simulink model "v01_aa_rii_16r4t11f_ver118sa"  (Simulink 7.6)
settings: Solver=ode45  StartTime=0.0  StopTime=128000  RelTol=1e-3
undefined symbols (not defined in the file or in its folder): sfp
libraries: xbsIndex_r4 x26962, monroe_library x154, xbsReplacements_r4 x78, simulink x32, dspsigops x32, casper_library_misc x30, xps_library x18
(part 22 of 176)

== system v01_aa_rii_16r4t11f_ver118sa/pfb_fir_real2/coeff_gen_0_15 ==
  sync : Inport
  Counter0 : xbsIndex_r4/Counter  cnt_type=Free Running  cnt_to=Inf  operation=Up  start_count=8  cnt_by_val=1  arith_type=Unsigned  n_bits=7  bin_pt=0  load_pin=off  rst=on  en=off  explicit_period=on  period=1  dbl_ovrd=off  use_behavioral_HDL=off  implementation=Fabric  block_type=counter
  Counter1 : xbsIndex_r4/Counter  cnt_type=Free Running  cnt_to=Inf  operation=Down  start_count=119  cnt_by_val=1  arith_type=Unsigned  n_bits=7  bin_pt=0  load_pin=off  rst=on  en=off  explicit_period=on  period=1  dbl_ovrd=off  use_behavioral_HDL=off  implementation=Fabric  block_type=counter
  Delay1 : xbsIndex_r4/Delay  rst=off  infoeditControl=Selection of Reset will increase slice count due to use of real FFs and instead of SRLs  en=off  latency=2  dbl_ovrd=off  reg_retiming=off  block_type=delay
  Delay2 : xbsIndex_r4/Delay  rst=off  infoeditControl=Selection of Reset will increase slice count due to use of real FFs and instead of SRLs  en=off  latency=1  dbl_ovrd=off  reg_retiming=off  block_type=delay
  Delay3 : xbsIndex_r4/Delay  rst=off  infoeditControl=Selection of Reset will increase slice count due to use of real FFs and instead of SRLs  en=off  latency=1  dbl_ovrd=off  reg_retiming=off  block_type=delay
  Delay4 : xbsIndex_r4/Delay  rst=off  infoeditControl=Selection of Reset will increase slice count due to use of real FFs and instead of SRLs  en=off  latency=2  dbl_ovrd=off  reg_retiming=off  block_type=delay
  coeff_ram_1 : xbsIndex_r4/Dual Port RAM  depth=128  initVector=[-9.76681628083641e-06 -0.000323674631039594 -0.000640685368821483 -0.000961464852068701 -0.00128668595239865 -0.00161702698554985 -0.00195317007673584 -0.00229579949852553 -0.00264559998341877 -0.00300325501333463 -0.00336944508827481 -0.00374484597646818 -0.0041301269483421 -0.00452594899670393 -0.00493296304555074 -0.00535180814995662 -0.00578310968951604 -0.00622747755784734 -0.00668550435068305 -0.00715776355509352 -0.00764480774240668 -0.00814716676740016 -0.00866534597635216 -0.00919982442654445 -0.00975105311981421 -0.0103194532527527 -0.0109054144861447 -0.011509293236238 -0.0121314109904214 -0.0127720526498779 -0.0134314649017638 -0.0141098546234445 -0.0148073873212959 -0.015524185606554 -0.0162603277106669 -0.0170158460425702 -0.0177907257902727 -0.0185849035690979 -0.0193982661188903 -0.0202306490524425 -0.0210818356573608 -0.0219515557535275 -0.0228394846082689 -0.0237452419112808 -0.0246683908113017 -0.0256084370164626 -0.0265648279601778 -0.0275369520343712 -0.0285241378917642 -0.0295256538188765 -0.0305407071813169 -0.0315684439428629 -0.0326079482597467 -0.0336582421514863 -0.0347182852495099 -0.0357869746247431 -0.0368631446952324 -0.0379455672147943 -0.0390329513435835 -0.0401239438013823 -0.0412171291043174 -0.0423110298856138 -0.0434041073008984 -0.0444947615184662 -0.0455813322948223 -0.0466620996357123 -0.0477352845427513 -0.0487990498456597 -0.0498515011200101 -0.0508906876902881 -0.0519146037179613 -0.0529211893741533 -0.0539083320964084 -0.0548738679289332 -0.0558155829455944 -0.0567312147548497 -0.0576184540856848 -0.0584749464535256 -0.0592982939049929 -0.0600860568402664 -0.0608357559117222 -0.0615448739974078 -0.0622108582478242 -0.0628311222043803 -0.0634030479877959 -0.0639239885546298 -0.0643912700200192 -0.0648021940446247 -0.0651540402836856 -0.0654440688960025 -0.06566952311058 -0.0658276318485765 -0.0659156123981315 -0.0659306731395561 -0.0658700163183022 -0.0657308408630492 -0.0655103452461749 -0.0652057303838135 -0.0648142025726343 -0.0643329764604135 -0.0637592780474138 -0.0630903477155274 -0.0623234432820887 -0.0614558430752086 -0.0604848490274413 -0.0594077897845465 -0.0582220238260738 -0.056924942594458 -0.0555139736292838 -0.0539865837033466 -0.0523402819571135 -0.0505726230281672 -0.0486812101721953 -0.0466636983720764 -0.0445177974316036 -0.0422412750503798 -0.0398319598764152 -0.0372877445329644 -0.0346065886161369 -0.0317865216598359 -0.0288256460645821 -0.0257221399868061 -0.0224742601852073 -0.0190803448208065 -0.0155388162073467 -0.011848183508731 -0.00800704538021982 -0.00401409255015518]  distributed_mem=Block RAM  init_a=0  init_b=0  rst_a=off  rst_b=off  en_a=off  en_b=off  latency=2  write_mode_A=No Read On Write  write_mode_B=No Read On Write  dbl_ovrd=off  optimize=Area  block_type=dpram
  coeff_ram_2 : xbsIndex_r4/Dual Port RAM  depth=128  initVector=[0.000131889660979235 0.00443201888730544 0.00888731333319452 0.013498689460842 0.0182669597527502 0.0231928305520307 0.0282768999833737 0.0335196559574605 0.0389214742615229 0.0444826167386781 0.0502032295585852 0.0560833415818892 0.0621228628208312 0.0683215829983153 0.0746791702076299 0.0811951696749281 0.0878690026264726 0.0946999652625533 0.10168722783988 0.108829833864151 0.116126699394389 0.123576612460526 0.131178232595614 0.138930090483912 0.146830587726002 0.154877996721945 0.163070460673406 0.171405993705518 0.179882481109162 0.188497679704198 0.197249218324085 0.206134598422161 0.215151194799783 0.224296256456348 0.233566907561132 0.242960148546725 0.252472857323742 0.262101790616336 0.271843585417932 0.28169476056646 0.291651718438258 0.301710746759654 0.311868020535164 0.322119604091054 0.332461453232956 0.342889417516048 0.353399242626224 0.363986572870548 0.374646953775156 0.385375834788674 0.396168572089086 0.407020431491882 0.417926591457195 0.428882146193547 0.439882108855684 0.450921414833902 0.461994925132158 0.473097429832141 0.48422365164041 0.495368249515587 0.506525822372513 0.517690912860181 0.528858011210183 0.540021559152312 0.551175953893889 0.562315552159312 0.573434674286248 0.584527608374814 0.595588614486041 0.606611928885839 0.617591768330647 0.628522334390862 0.639397817808129 0.650212402882503 0.660960271885469 0.671635609494744 0.682232607246797 0.692745468002935 0.703168410424828 0.713495673455308 0.723721520800239 0.733840245407289 0.743846173937386 0.753733671224655 0.763497144720641 0.773131048918607 0.782629889753732 0.791988228975025 0.801200688484807 0.810261954641631 0.819166782522532 0.827910000140534 0.836486512613386 0.844891306279528 0.853119452757334 0.861166112943733 0.869026540948364 0.876696087959463 0.884170206037768 0.891444451834754 0.898514490231627 0.905376097895536 0.912025166749558 0.9184577073531 0.924669852189424 0.930657858857099 0.936418113162282 0.941947132108819 0.947241566783232 0.952298205131806 0.957113974627046 0.961685944820906 0.966011329782295 0.970087490416478 0.9739119366641 0.977482329577674 0.980796483273521 0.983852366757218 0.986648105620789 0.989181983609969 0.991452444059991 0.993458091198508 0.995197691314345 0.996670173790967 0.997874632003604 0.998810324079201 0.999476673518401 0.999873269678985]  distributed_mem=Block RAM  init_a=0  init_b=0  rst_a=off  rst_b=off  en_a=off  en_b=off  latency=2  write_mode_A=No Read On Write  write_mode_B=No Read On Write  dbl_ovrd=off  optimize=Area  block_type=dpram
  coeff_ram_3 : xbsIndex_r4/Dual Port RAM  depth=128  initVector=[0.999999868120266 0.999856390808123 0.999442926180455 0.998759729072997 0.997807220505575 0.996585987329003 0.995096781732979 0.993340520615446 0.99131828481406 0.989031318200503 0.986481026638539 0.983668976806834 0.980596894887697 0.977266665123027 0.973680328238889 0.969840079740251 0.96574826807758 0.961407392687059 0.956820101906379 0.95198919076813 0.946917598672946 0.941608406944702 0.936064836270128 0.930290244025361 0.924288121492039 0.918062090965654 0.91161590275898 0.904953432103512 0.898078675951906 0.890995749684561 0.883708883723521 0.876222420057003 0.868540808677901 0.860668603939747 0.852610460833631 0.844371131189694 0.835955459806863 0.827368380514556 0.818614912170158 0.809700154596117 0.800629284460566 0.791407551105419 0.782040272325951 0.772532830105885 0.762890666312066 0.753119278352835 0.743224214804217 0.733211071008104 0.723085484646579 0.712853131296598 0.702519719969201 0.692090988637482 0.681572699757496 0.670970635786334 0.660290594701535 0.649538385526024 0.638719823862754 0.627840727443177 0.616906911693676 0.605924185324051 0.5948983459421 0.583835175698334 0.572740436964789 0.561619868051874 0.550479178967135 0.539324047219761 0.528160113674606 0.516992978459435 0.50582819692903 0.494671275689747 0.48352766868801 0.472402773366174 0.461301926889109 0.45023040244476 0.439193405621868 0.428196070867941 0.417243458030464 0.406340548984262 0.39549224434781 0.384703360291205 0.373978625438387 0.363322677866115 0.35274006220209 0.342235226824484 0.331812521165061 0.321476193117935 0.311230386555891 0.301079138956108 0.291026379136963 0.281075925107508 0.271231482031073 0.26149664030433 0.25187487375303 0.24236953794549 0.232983868624808 0.223720980260621 0.214583864721127 0.205575390065943 0.196698299460255 0.187955210210599 0.179348612922444 0.170880870779681 0.162554218945948 0.154370764087607 0.146332484018087 0.138441227463148 0.130698713946519 0.123106533795237 0.115666148263892 0.108378889776859 0.101245962287486 0.0942684417530879 0.087447276724481 0.0807832890486749 0.0742771746832388 0.0679295046207398 0.0617407259215459 0.0557111628531857 0.0498410181343505 0.0441303742815262 0.0385791950561456 0.0331873270100564 0.0279545011270101 0.022880334557785 0.0179643324464767 0.0132058898453996 0.00860429371596978 0.00415872501285985]  distributed_mem=Block RAM  init_a=0  init_b=0  rst_a=off  rst_b=off  en_a=off  en_b=off  latency=2  write_mode_A=No Read On Write  write_mode_B=No Read On Write  dbl_ovrd=off  optimize=Area  block_type=dpram
  coeff_ram_4 : xbsIndex_r4/Dual Port RAM  depth=128  initVector=[-0.000131739151355809 -0.00426812326391606 -0.00825155109486444 -0.0120832433082974 -0.0157645150049071 -0.0192967731989124 -0.0226815142325243 -0.0259203211311373 -0.0290148609024857 -0.0319668817830422 -0.0347782104349725 -0.0374507490969939 -0.0399864726925128 -0.0423874258984425 -0.044655720178123 -0.0467935307817807 -0.0488030937179814 -0.0506867026995366 -0.0524467060673303 -0.0540855036955342 -0.0556055438816755 -0.0570093202250184 -0.0582993684967072 -0.0594782635051042 -0.0605486159597422 -0.0615130693372847 -0.0623742967528647 -0.0631349978401424 -0.0637978956433909 -0.0643657335248817 -0.064841272090801 -0.0652272861388886 -0.0655265616309388 -0.0657418926932587 -0.0658760786481207 -0.0659319210791943 -0.0659122209338789 -0.0658197756654005 -0.0656573764174654 -0.0654278052542005 -0.0651338324380346 -0.0647782137581042 -0.0643636879116891 -0.0638929739411062 -0.063368768728406 -0.0627937445501364 -0.0621705466943507 -0.0615017911419477 -0.0607900623143467 -0.0600379108894042 -0.0592478516873886 -0.058422361628734 -0.0575638777651976 -0.0566747953859491 -0.055757466200019 -0.0548141965964363 -0.0538472459832827 -0.0528588252067891 -0.0518510950515002 -0.0508261648224251 -0.0497860910099933 -0.0487328760385286 -0.0476684670988495 -0.0465947550655016 -0.0455135734990219 -0.0444266977335309 -0.0433358440498461 -0.0422426689342039 -0.0411487684225764 -0.0400556775304669 -0.0389648697679635 -0.0378777567397334 -0.0367956878295371 -0.035719949968745 -0.0346517674882394 -0.0335923020529909 -0.0325426526785005 -0.0315038558282083 -0.0304768855908741 -0.0294626539368496 -0.0284620110520695 -0.0274757457485057 -0.0265045859497419 -0.0255491992502456 -0.0246101935468339 -0.0236881177407513 -0.0227834625087025 -0.0218966611411123 -0.02102809044581 -0.0201780717152727 -0.0193468717554942 -0.0185347039744836 -0.0177417295283403 -0.016968058522793 -0.0162137512680375 -0.0154788195846594 -0.0147632281583751 -0.0140668959412854 -0.0133896975972904 -0.0127314649892776 -0.0120919887056604 -0.0114710196238096 -0.0108682705078952 -0.0102834176386267 -0.00971610247236133 -0.00916593332702641 -0.00863248709229016 -0.00811531096140056 -0.00761392418210336 -0.00712781982404429 -0.00665646656005785 -0.00619931045874612 -0.00575577678575429 -0.00532527181115724 -0.00490718462038177 -0.00450088892610224 -0.00410574487856475 -0.0037211008718141 -0.00334629534332111 -0.00298065856453372 -0.00262351441990408 -0.00227418217197576 -0.00193197821015024 -0.00159621778078896 -0.001266216696348 -0.000941293021285331 -0.000620768732526511 -0.000303971352322718]  distributed_mem=Block RAM  init_a=0  init_b=0  rst_a=off  rst_b=off  en_a=off  en_b=off  latency=2  write_mode_A=No Read On Write  write_mode_B=No Read On Write  dbl_ovrd=off  optimize=Area  block_type=dpram
  const_din : xbsIndex_r4/Constant  const=0  gui_display_data_type=Fixed-point  arith_type=Signed (2's comp)  n_bits=18  bin_pt=17  preci_type=Single  exp_width=8  frac_width=24  explicit_period=on  period=1  equ=P=C  opselect=C  inp2=PCIN>>17  opr=+  inp1=P  carry=CIN  dbl_ovrd=off  block_type=constant
  const_we : xbsIndex_r4/Constant  const=0  gui_display_data_type=Fixed-point  arith_type=Boolean  n_bits=16  bin_pt=14  preci_type=Single  exp_width=8  frac_width=24  explicit_period=on  period=1  equ=P=C  opselect=C  inp2=PCIN>>17  opr=+  inp1=P  carry=CIN  dbl_ovrd=off  block_type=constant
  cram_lower : Subsystem
  cram_upper : Subsystem
  sync_out : Outport
  coeff : Outport  Port=2
  coeff_rev : Outport  Port=3
  wires:
  sync/1 -> Counter1/1, Counter0/1, sync_out/1
  Counter0/1 -> coeff_ram_4/1, coeff_ram_3/1, Delay2/1, Delay1/1
  const_din/1 -> coeff_ram_4/5, coeff_ram_4/2, coeff_ram_3/5, coeff_ram_3/2, coeff_ram_2/5, coeff_ram_2/2, coeff_ram_1/5, coeff_ram_1/2
  const_we/1 -> coeff_ram_4/6, coeff_ram_4/3, coeff_ram_3/6, coeff_ram_3/3, coeff_ram_2/6, coeff_ram_2/3, coeff_ram_1/6, coeff_ram_1/3
  Counter1/1 -> Delay4/1, Delay3/1, coeff_ram_2/4, coeff_ram_1/4
  cram_lower/1(out) -> coeff_rev/1
  cram_upper/1(out) -> coeff/1
  coeff_ram_1/2 -> cram_lower/4(in3)
  coeff_ram_2/2 -> cram_lower/3(in2)
  coeff_ram_3/2 -> cram_lower/2(in1)
  coeff_ram_4/2 -> cram_lower/1(in0)
  coeff_ram_4/1 -> cram_upper/4(in3)
  coeff_ram_3/1 -> cram_upper/3(in2)
  coeff_ram_2/1 -> cram_upper/2(in1)
  coeff_ram_1/1 -> cram_upper/1(in0)
  Delay4/1 -> coeff_ram_4/4
  Delay3/1 -> coeff_ram_3/4
  Delay2/1 -> coeff_ram_2/1
  Delay1/1 -> coeff_ram_1/1

== system v01_aa_rii_16r4t11f_ver118sa/pfb_fir_real2/coeff_gen_1_14 ==
  sync : Inport
  Counter0 : xbsIndex_r4/Counter  cnt_type=Free Running  cnt_to=Inf  operation=Up  start_count=8  cnt_by_val=1  arith_type=Unsigned  n_bits=7  bin_pt=0  load_pin=off  rst=on  en=off  explicit_period=on  period=1  dbl_ovrd=off  use_behavioral_HDL=off  implementation=Fabric  block_type=counter
  Counter1 : xbsIndex_r4/Counter  cnt_type=Free Running  cnt_to=Inf  operation=Down  start_count=119  cnt_by_val=1  arith_type=Unsigned  n_bits=7  bin_pt=0  load_pin=off  rst=on  en=off  explicit_period=on  period=1  dbl_ovrd=off  use_behavioral_HDL=off  implementation=Fabric  block_type=counter
  Delay6 : xbsIndex_r4/Delay  rst=off  infoeditControl=Selection of Reset will increase slice count due to use of real FFs and instead of SRLs  en=off  latency=2  dbl_ovrd=off  reg_retiming=off  block_type=delay
  Delay7 : xbsIndex_r4/Delay  rst=off  infoeditControl=Selection of Reset will increase slice count due to use of real FFs and instead of SRLs  en=off  latency=1  dbl_ovrd=off  reg_retiming=off  block_type=delay
  Delay8 : xbsIndex_r4/Delay  rst=off  infoeditControl=Selection of Reset will increase slice count due to use of real FFs and instead of SRLs  en=off  latency=1  dbl_ovrd=off  reg_retiming=off  block_type=delay
  Delay9 : xbsIndex_r4/Delay  rst=off  infoeditControl=Selection of Reset will increase slice count due to use of real FFs and instead of SRLs  en=off  latency=2  dbl_ovrd=off  reg_retiming=off  block_type=delay
  coeff_ram_1 : xbsIndex_r4/Dual Port RAM  depth=128  initVector=[-2.93076314848341e-05 -0.0003433900277522 -0.000660616724491361 -0.000981654031152269 -0.00130717520695048 -0.00163785885390256 -0.00197438728040023 -0.00231744483744514 -0.00266771622971605 -0.00302588480368873 -0.00339263081507411 -0.00376862967788268 -0.00415455019746389 -0.00455105278990572 -0.00495878769021458 -0.00537839315172707 -0.00581049363923343 -0.00625569801831852 -0.00671459774344815 -0.00718776504734843 -0.00767575113424205 -0.00817908437951815 -0.00869826853842296 -0.00923378096636465 -0.00978607085342968 -0.0103555574757079 -0.0109426284660206 -0.0115476381066404 -0.0121709056465795 -0.0128127136460145 -0.0134733063503947 -0.0141528880967647 -0.0148516217548094 -0.0155696272051008 -0.0163069798569994 -0.0170637092086298 -0.0178397974513133 -0.0186351781208041 -0.0194497347976292 -0.0202832998587928 -0.021135653283053 -0.0220065215119295 -0.0228955763685474 -0.0238024340363641 -0.0247266540997661 -0.0256677386484617 -0.0266251314475281 -0.0275982171749041 -0.0285863207280505 -0.0295887066014229 -0.0306045783363319 -0.0316330780446816 -0.0326732860080024 -0.0337242203531073 -0.0347848368056193 -0.0358540285225293 -0.0369306260048564 -0.0380133970913911 -0.0391010470344118 -0.0401922186581682 -0.0412854926008334 -0.042379387640529 -0.0434723611059268 -0.0445628093718371 -0.0456490684400887 -0.0467294146059067 -0.0478020652098928 -0.0488651794756097 -0.0499168594326669 -0.0509551509251039 -0.0519780447047611 -0.0529834776092245 -0.0539693338238275 -0.0549334462270865 -0.0558735978188449 -0.0567875232302953 -0.057672910314946 -0.0585274018194948 -0.0593485971334722 -0.0601340541164121 -0.0608812910012097 -0.0615877883722247 -0.062250991216591 -0.0628683110470947 -0.0634371280948897 -0.0639547935702203 -0.0644186319892339 -0.0648259435648696 -0.065174006659724 -0.065460080298705 -0.0656814067392009 -0.0658352140964069 -0.0659187190213748 -0.065929129429266 -0.0658636472752194 -0.0657194713751676 -0.065493800268865 -0.0651838351223255 -0.0647867826667992 -0.0642998581713582 -0.063720288446101 -0.0630453148729286 -0.0622721964607946 -0.0613982129222802 -0.0604206677682987 -0.0593368914176931 -0.0581442443184503 -0.056840120077217 -0.0554219485937764 -0.0538871991971081 -0.0522333837796366 -0.0504580599262472 -0.0485588340346322 -0.0465333644235189 -0.0443793644253177 -0.0420946054597251 -0.0396769200848134 -0.0371242050221413 -0.0344344241524247 -0.0316056114783179 -0.0286358740508674 -0.0255233948562196 -0.0222664356591845 -0.0188633398002829 -0.0153125349429337 -0.0116125357674718 -0.00776194660872291 -0.00375946403390469]  distributed_mem=Block RAM  init_a=0  init_b=0  rst_a=off  rst_b=off  en_a=off  en_b=off  latency=2  write_mode_A=No Read On Write  write_mode_B=No Read On Write  dbl_ovrd=off  optimize=Area  block_type=dpram
  coeff_ram_2 : xbsIndex_r4/Dual Port RAM  depth=128  initVector=[0.000396120642335426 0.00470591890888301 0.00917094267820741 0.0137921019785327 0.0185702027210988 0.0235059445454233 0.0285999187454048 0.0338526062790413 0.0392643758644625 0.0448354821648979 0.0505660640651239 0.0564561430418494 0.062505621630413 0.068714281990078 0.0750817845701153 0.0816076668787734 0.0882913423571361 0.0951320993597678 0.102129100243943 0.109281380569157 0.116587848408492 0.124047283773336 0.131658338152792 0.139419534169051 0.147329265349853 0.155385796019061 0.163587261306239 0.17193166727603 0.180416891177976 0.189040681817328 0.197800660047261 0.206694319382768 0.215719026736416 0.224872023275984 0.234150425403911 0.24355122585832 0.253071294935296 0.262707381831929 0.272456116109539 0.282314009276356 0.292277456488801 0.302342738370398 0.312506022947209 0.322763367698576 0.333110721721806 0.343543928009345 0.354058725836823 0.36465075326028 0.375315549720722 0.386048558754067 0.396845130804404 0.407700526138397 0.418609917858536 0.429568395012833 0.440570965798452 0.45161256085667 0.462688036656438 0.473792178963735 0.484919706393793 0.496065274043196 0.50722347719874 0.518388855119869 0.529555894891419 0.540719035343303 0.551872671033708 0.563011156292289 0.574128809319776 0.58521991634035 0.59627873580306 0.607299502628509 0.618276432496971 0.629203726174062 0.64007557387001 0.650886159628549 0.661629665741423 0.672300277184415 0.682892186070835 0.693399596118318 0.703816727124803 0.714137819449515 0.724357138494772 0.734468979184421 0.744467670434693 0.754347579613286 0.764103116982464 0.77372874012197 0.783218958327578 0.792568336981106 0.801771501887729 0.810823143576478 0.819718021559813 0.8284509685482 0.837016894615666 0.845410791312332 0.853627735719985 0.861662894446779 0.869511527557234 0.877168992433738 0.884630747565818 0.89189235626354 0.898949490291422 0.905797933419361 0.912433584887118 0.918852462779008 0.925050707305512 0.931024583988624 0.936770486747836 0.942284940883741 0.947564605956374 0.952606278555451 0.957406894959828 0.961963533683572 0.966273417906152 0.970333917784379 0.974142552643838 0.977696993047649 0.980995062740551 0.984034740466392 0.986814161657246 0.989331619992497 0.99158556882637 0.993574622482497 0.995297557414254 0.996753313229722 0.997940993580283 0.998859866911946 0.999509367078706 0.999889093817292]  distributed_mem=Block RAM  init_a=0  init_b=0  rst_a=off  rst_b=off  en_a=off  en_b=off  latency=2  write_mode_A=No Read On Write  write_mode_B=No Read On Write  dbl_ovrd=off  optimize=Area  block_type=dpram
  coeff_ram_3 : xbsIndex_r4/Dual Port RAM  depth=128  initVector=[0.99999881308287 0.99983845724535 0.999408125146119 0.998708082015126 0.997738759248423 0.996500754046364 0.994994828912819 0.993221911015907 0.991183091410863 0.988879624125808 0.986312925111323 0.983484571054852 0.980396298061105 0.977050000199752 0.973447727921836 0.969591686346453 0.965484233419392 0.961127877945523 0.956525277496874 0.951679236198442 0.946592702393901 0.9412687661935 0.935710656906542 0.929921740360949 0.923905516112543 0.91766561454676 0.911205793875616 0.904529937032865 0.897642048470364 0.89054625085876 0.883246781695714 0.875747989824948 0.868054331869494 0.860170368582608 0.852100761119866 0.843850267236071 0.83542373741062 0.826826110905088 0.818062411756812 0.809137744712344 0.800057291104675 0.790826304678184 0.781450107365311 0.771934085019004 0.762283683105003 0.752504402358078 0.742601794406352 0.732581457367859 0.722449031423529 0.712210194370772 0.701870657161869 0.69143615943137 0.680912465016712 0.670305357476243 0.659620635608864 0.648864108979451 0.638041593454237 0.627158906750289 0.616221864003197 0.605236273357073 0.594207931580896 0.583142619715245 0.572046098753371 0.560924105360555 0.549782347635614 0.538626500918398 0.527462203647025 0.516295053268579 0.505130602206887 0.493974353890972 0.482831758847658 0.471708210861755 0.460609043207173 0.449539524952209 0.438504857342202 0.427510170262607 0.416560518785519 0.405660879802503 0.394816148746565 0.384031136405928 0.373310565832237 0.362659069345662 0.352081185639287 0.341581356985059 0.331163926543447 0.32083313577887 0.310593121982802 0.30044791590639 0.290401439504255 0.280457503791059 0.270619806812289 0.260891931730578 0.251277345028768 0.241779394830796 0.232401309341353 0.223146195405141 0.214017037186428 0.205016694969482 0.196147904080311 0.187413273930046 0.178815287180153 0.170356299029535 0.162038536623461 0.153864098584149 0.145834954662675 0.137952945511775 0.13021978257899 0.122637048119462 0.115206195327575 0.107928548586534 0.100805303834826 0.0938375290484057 0.0870261648373483 0.0803720251555647 0.0738757981220955 0.0675380469523722 0.0613592109977351 0.0553396068913899 0.049479429798884 0.0437787547710844 0.0382375381975392 0.032855619358016 0.027632722069913 0.022568456429154 0.0176623206420921 0.012913702945863 0.00832188361455311 0.0038860370484663]  distributed_mem=Block RAM  init_a=0  init_b=0  rst_a=off  rst_b=off  en_a=off  en_b=off  latency=2  write_mode_A=No Read On Write  write_mode_B=No Read On Write  dbl_ovrd=off  optimize=Area  block_type=dpram
  coeff_ram_4 : xbsIndex_r4/Dual Port RAM  depth=128  initVector=[-0.000394766056293522 -0.0045215564607811 -0.00849546406162938 -0.0123177154979143 -0.0159896316895057 -0.0195126253100011 -0.022888198197393 -0.0261179387056621 -0.0292035190005407 -0.032146692302723 -0.0349492900818404 -0.0376132192045508 -0.0401404590401191 -0.0425330585268906 -0.044793133203081 -0.0469228622053225 -0.0489244852384181 -0.0508002995197669 -0.0525526567019253 -0.0541839597767744 -0.0556966599647567 -0.0570932535926411 -0.0583762789632651 -0.0595483132206868 -0.0606119692141633 -0.0615698923643488 -0.0624247575350805 -0.0631792659140917 -0.0638361419059583 -0.0643981300405479 -0.0648679919002013 -0.0652485030688332 -0.0655424501060908 -0.0657526275496605 -0.0658818349487574 -0.0659328739317782 -0.0659085453110368 -0.0658116462274383 -0.0656449673378855 -0.065411290048137 -0.0651133837937727 -0.0647540033718402 -0.0643358863256872 -0.0638617503853992 -0.0633342909661855 -0.0627561787269704 -0.0621300571913608 -0.0614585404330756 -0.0607442108278302 -0.05998961687358 -0.0591972710809313 -0.0583696479354368 -0.0575091819333925 -0.0566182656926582 -0.0556992481399241 -0.0547544327757455 -0.0537860760185674 -0.0527963856288598 -0.0517875192143797 -0.0507615828174742 -0.0497206295852371 -0.0486666585232234 -0.0476016133333257 -0.0465273813363107 -0.0454457924794083 -0.0443586184292448 -0.0432675717503049 -0.0421743051690054 -0.0410804109233585 -0.039987420198103 -0.038896802645078 -0.0378099659885123 -0.0367282557148054 -0.0356529548462741 -0.0345852837982445 -0.0335264003187688 -0.0324773995101554 -0.0314393139314059 -0.0304131137805592 -0.0293997071558562 -0.0283999403945475 -0.0274145984880831 -0.0264444055723349 -0.0254900254914268 -0.0245520624336598 -0.0236310616379493 -0.0227275101691114 -0.0218418377602643 -0.0209744177205413 -0.020125567906243 -0.0192955517534914 -0.0184845793703889 -0.0176928086866212 -0.016920346658392 -0.0161672505265193 -0.0154335291254751 -0.0147191442411025 -0.014024012014698 -0.013348004391108 -0.0126909506084473 -0.012052638727016 -0.0114328171949543 -0.010831196448152 -0.0102474505418992 -0.00968121881174424 -0.00913210756100777 -0.0085996917723833 -0.00808351684104443 -0.00758310032666915 -0.00709793372178607 -0.00662748423384496 -0.00617119657841499 -0.00572849478091779 -0.00529878398431046 -0.00488145226014341 -0.00447587242043229 -0.00408140382779974 -0.00369739420136302 -0.00332318141586631 -0.00295809529158295 -0.00260145937254169 -0.00225259269066331 -0.00191081151342877 -0.00157543107273786 -0.00124576727265792 -0.00092113837380536 -0.000600866652148824 -0.000284280030071002]  distributed_mem=Block RAM  init_a=0  init_b=0  rst_a=off  rst_b=off  en_a=off  en_b=off  latency=2  write_mode_A=No Read On Write  write_mode_B=No Read On Write  dbl_ovrd=off  optimize=Area  block_type=dpram
  const_din : xbsIndex_r4/Constant  const=0  gui_display_data_type=Fixed-point  arith_type=Signed (2's comp)  n_bits=18  bin_pt=17  preci_type=Single  exp_width=8  frac_width=24  explicit_period=on  period=1  equ=P=C  opselect=C  inp2=PCIN>>17  opr=+  inp1=P  carry=CIN  dbl_ovrd=off  block_type=constant
  const_we : xbsIndex_r4/Constant  const=0  gui_display_data_type=Fixed-point  arith_type=Boolean  n_bits=16  bin_pt=14  preci_type=Single  exp_width=8  frac_width=24  explicit_period=on  period=1  equ=P=C  opselect=C  inp2=PCIN>>17  opr=+  inp1=P  carry=CIN  dbl_ovrd=off  block_type=constant
  cram_lower : Subsystem
  cram_upper : Subsystem
  sync_out : Outport
  coeff : Outport  Port=2
  coeff_rev : Outport  Port=3
  wires:
  sync/1 -> Counter1/1, Counter0/1, sync_out/1
  Counter0/1 -> coeff_ram_4/1, coeff_ram_3/1, Delay7/1, Delay6/1
  const_din/1 -> coeff_ram_4/5, coeff_ram_4/2, coeff_ram_3/5, coeff_ram_3/2, coeff_ram_2/5, coeff_ram_2/2, coeff_ram_1/5, coeff_ram_1/2
  const_we/1 -> coeff_ram_4/6, coeff_ram_4/3, coeff_ram_3/6, coeff_ram_3/3, coeff_ram_2/6, coeff_ram_2/3, coeff_ram_1/6, coeff_ram_1/3
  Counter1/1 -> Delay9/1, Delay8/1, coeff_ram_2/4, coeff_ram_1/4
  cram_lower/1(out) -> coeff_rev/1
  cram_upper/1(out) -> coeff/1
  coeff_ram_1/2 -> cram_lower/4(in3)
  coeff_ram_2/2 -> cram_lower/3(in2)
  coeff_ram_3/2 -> cram_lower/2(in1)
  coeff_ram_4/2 -> cram_lower/1(in0)
  coeff_ram_4/1 -> cram_upper/4(in3)
  coeff_ram_3/1 -> cram_upper/3(in2)
  coeff_ram_2/1 -> cram_upper/2(in1)
  coeff_ram_1/1 -> cram_upper/1(in0)
  Delay9/1 -> coeff_ram_4/4
  Delay8/1 -> coeff_ram_3/4
  Delay7/1 -> coeff_ram_2/1
  Delay6/1 -> coeff_ram_1/1

== system v01_aa_rii_16r4t11f_ver118sa/pfb_fir_real2/coeff_gen_2_13 ==
  sync : Inport
  Counter0 : xbsIndex_r4/Counter  cnt_type=Free Running  cnt_to=Inf  operation=Up  start_count=8  cnt_by_val=1  arith_type=Unsigned  n_bits=7  bin_pt=0  load_pin=off  rst=on  en=off  explicit_period=on  period=1  dbl_ovrd=off  use_behavioral_HDL=off  implementation=Fabric  block_type=counter
  Counter1 : xbsIndex_r4/Counter  cnt_type=Free Running  cnt_to=Inf  operation=Down  start_count=119  cnt_by_val=1  arith_type=Unsigned  n_bits=7  bin_pt=0  load_pin=off  rst=on  en=off  explicit_period=on  period=1  dbl_ovrd=off  use_behavioral_HDL=off  implementation=Fabric  block_type=counter
  Delay11 : xbsIndex_r4/Delay  rst=off  infoeditControl=Selection of Reset will increase slice count due to use of real FFs and instead of SRLs  en=off  latency=2  dbl_ovrd=off  reg_retiming=off  block_type=delay
  Delay12 : xbsIndex_r4/Delay  rst=off  infoeditControl=Selection of Reset will increase slice count due to use of real FFs and instead of SRLs  en=off  latency=1  dbl_ovrd=off  reg_retiming=off  block_type=delay
  Delay13 : xbsIndex_r4/Delay  rst=off  infoeditControl=Selection of Reset will increase slice count due to use of real FFs and instead of SRLs  en=off  latency=1  dbl_ovrd=off  reg_retiming=off  block_type=delay
  Delay14 : xbsIndex_r4/Delay  rst=off  infoeditControl=Selection of Reset will increase slice count due to use of real FFs and instead of SRLs  en=off  latency=2  dbl_ovrd=off  reg_retiming=off  block_type=delay
  coeff_ram_1 : xbsIndex_r4/Dual Port RAM  depth=128  initVector=[-4.88581563770449e-05 -0.000363117704122796 -0.000680562963101438 -0.00100186072361597 -0.00132768462620228 -0.00165871355276197 -0.00199562998836516 -0.00233911835584811 -0.00268986332538073 -0.00304854810122696 -0.00341585268796654 -0.00379245213848867 -0.00417901478610864 -0.00457620046319489 -0.00498465870872888 -0.00540502696725076 -0.0058379287816729 -0.006283971982468 -0.00674374687576157 -0.00721782443287702 -0.00770675448389841 -0.00821106391782825 -0.00873125489192799 -0.009267803052835 -0.00982115577205335 -0.0103917303984157 -0.01097991253011 -0.0115860543088596 -0.0122104727388325 -0.0128534480328467 -0.0135152219884182 -0.0141959963961807 -0.014895931483183 -0.0156151443935425 -0.0163537077089065 -0.0171116480111361 -0.0178889444895964 -0.018685527595394 -0.019501277744862 -0.0203360240745485 -0.0211895432499133 -0.0220615583298899 -0.0229517376894121 -0.0238596940019506 -0.0247849832840408 -0.0257271040037258 -0.0266854962547664 -0.0276595409984077 -0.0286485593744174 -0.0296518120830377 -0.0306684988394198 -0.0316977579020283 -0.0327386656764239 -0.0337902363957518 -0.034851421879175 -0.0359211113694085 -0.036998131450419 -0.0380812460462669 -0.0391691565019729 -0.040260501747199 -0.0413538585434383 -0.0424477418153125 -0.0435406050664742 -0.0446308408805181 -0.0457167815071996 -0.0467966995341607 -0.0478688086442617 -0.0489312644585134 -0.049982165464501 -0.0510195540300896 -0.0520414175020933 -0.0530456893894908 -0.0540302506306603 -0.0549929309440069 -0.0559315102612497 -0.0568437202425312 -0.0577272458724093 -0.058579727135689 -0.059398760771948 -0.0601819021075101 -0.060926666963517 -0.061630533638654 -0.0622909449649808 -0.0629053104352271 -0.0634710083998119 -0.0639853883317551 -0.0644457731575542 -0.0648494616520091 -0.0651937308948896 -0.0654758387872513 -0.065693026625122 -0.065842521728196 -0.0659215401210952 -0.0659272892646755 -0.0658569708347825 -0.0657077835457865 -0.0654769260161574 -0.0651615996732698 -0.0647590116945673 -0.0642663779821502 -0.0636809261677927 -0.0629998986453401 -0.0622205556273847 -0.0613401782230671 -0.0603560715338077 -0.0592655677637264 -0.0580660293414729 -0.0567548520501512 -0.055329468161994 -0.0537873495744097 -0.052126010944004 -0.0503430128151544 -0.0484359647397008 -0.0464025283843011 -0.0442404206219902 -0.0419474166044778 -0.039521352811716 -0.0369601300752709 -0.034261716572039 -0.0314241507848585 -0.0284455444265786 -0.0253240853241706 -0.0220580402594822 -0.0186457577632666 -0.0150856708591427 -0.0113762997541813 -0.00751625447284484 -0.00350423743105158]  distributed_mem=Block RAM  init_a=0  init_b=0  rst_a=off  rst_b=off  en_a=off  en_b=off  latency=2  write_mode_A=No Read On Write  write_mode_B=No Read On Write  dbl_ovrd=off  optimize=Area  block_type=dpram
  coeff_ram_2 : xbsIndex_r4/Dual Port RAM  depth=128  initVector=[0.000660954052819527 0.00498042531311985 0.00945518196140141 0.0140861275831942 0.0188740615100578 0.0238196766698134 0.0289235575177928 0.0341861780518729 0.0396078999139903 0.0451889705807531 0.0509295216456887 0.0568295671955794 0.0628890022832569 0.0691076014991322 0.075485017643649 0.0820207805027518 0.0887142957283631 0.0955648438257635 0.102571579249665 0.109733529610668 0.11704959499367 0.124518547389712 0.132139030242602 0.139909558111574 0.147828516451102 0.155894161508883 0.164104620342888 0.172457890958243 0.180951842564601 0.189584215954528 0.198352624003312 0.207254552290474 0.216287359843142 0.225448280001303 0.23473442140486 0.244142769102244 0.253670185780255 0.263313413114637 0.273069073240789 0.282933670343884 0.292903592367536 0.302975112840019 0.313144392816958 0.323407482939224 0.333760325604698 0.34419875725242 0.354718510757511 0.365315217935164 0.375984412151841 0.38672153104174 0.39752191932644 0.408380831735554 0.419293436026074 0.430254816098017 0.441259975203838 0.452303839249013 0.463381260181041 0.474487019464064 0.48561583163618 0.496762347946422 0.507921160068316 0.519086803886809 0.530253763355295 0.541416474419366 0.552569329003871 0.563706679059733 0.574822840666972 0.585912098190244 0.596968708483206 0.607986905137903 0.618960902775346 0.629884901373399 0.640753090628017 0.65155965434386 0.662298774850259 0.672964637438462 0.68355143481607 0.694053371574532 0.704464668665557 0.714779567882276 0.724992336340957 0.735097270959087 0.745088702925623 0.754961002159193 0.764708581750057 0.774325902381622 0.78380747672733 0.79314787381875 0.802341723380712 0.811383720129364 0.820268628029058 0.828991284503973 0.83754660460048 0.845929585096226 0.854135308552013 0.862158947302577 0.869995767382404 0.877641132382832 0.88509050723669 0.892339461926826 0.899383675114945 0.906218937687225 0.912841156213295 0.919246356315196 0.925430685943074 0.931390418554406 0.937121956193666 0.942621832469449 0.947886715426117 0.952913410307209 0.957698862207882 0.962240158613806 0.96653453182404 0.970579361255507 0.97437217562681 0.97791065501927 0.981192632813148 0.98421609749716 0.986979194349518 0.989480226988835 0.991717658793384 0.993690114187311 0.995396379792542 0.996835405445253 0.998006305075904 0.998908357451973 0.999541006782655 0.999903863184942]  distributed_mem=Block RAM  init_a=0  init_b=0  rst_a=off  rst_b=off  en_a=off  en_b=off  latency=2  write_mode_A=No Read On Write  write_mode_B=No Read On Write  dbl_ovrd=off  optimize=Area  block_type=dpram
  coeff_ram_3 : xbsIndex_r4/Dual Port RAM  depth=128  initVector=[0.999996703010618 0.999819469033848 0.99937227049918 0.99865538302991 0.997669248396904 0.996414474148094 0.99489183309903 0.99310226268497 0.991046864175156 0.988726901750045 0.986143801442402 0.983299149943296 0.980194693274162 0.976832335326256 0.973214136268905 0.96934231082814 0.965219226437387 0.960847401262029 0.956229502099781 0.951368342158929 0.946266878716606 0.940928210659408 0.935355575908736 0.929552348733396 0.923522036952073 0.917268279028415 0.910794841061549 0.904105613674977 0.897204608806863 0.890095956404854 0.882783901028629 0.875272798363482 0.867567111648328 0.859671408021581 0.85159035478845 0.843328715613261 0.834891346640481 0.826283192548187 0.817509282537785 0.808574726263826 0.799484709707849 0.790244491000197 0.780859396193804 0.771334814994015 0.761676196448496 0.751889044601353 0.741978914115602 0.731951405868127 0.721812162521336 0.711566864075661 0.701221223407151 0.690780981794317 0.680251904438464 0.669639775981697 0.658950396026799 0.648189574663153 0.63736312800289 0.626476873731375 0.615536626676177 0.604548194398595 0.593517372811782 0.582449941829514 0.571351661049535 0.560228265475438 0.549085461280935 0.537928921620352 0.526764282489097 0.515597138637818 0.504433039543876 0.493277485443695 0.482135923429498 0.471013743613828 0.459916275365199 0.448848783618133 0.437816465260744 0.426824445602949 0.415877774928295 0.404981425132285 0.394140286450004 0.383359164275727 0.372642776077104 0.361995748406401 0.351422614011174 0.340927809046632 0.330515670391855 0.320190433071889 0.30995622778765 0.299817078555439 0.289776900457747 0.279839497506921 0.270008560623129 0.260287665727943 0.250680271954739 0.241189719976977 0.231819230455313 0.222571902604355 0.213450712879759 0.204458513786229 0.195598032806856 0.18687187145411 0.178282504442668 0.169832278984131 0.161523414203573 0.153358000677705 0.14533800009436 0.137465245032836 0.129741438864538 0.122168155773222 0.114746840894046 0.107478810570477 0.100365252728021 0.0934072273636063 0.0866056671493396 0.0799613781492418 0.0734750406474625 0.0671472100863593 0.0609783181127218 0.0549686737303183 0.049118464556838 0.0434277581832066 0.037896503633149 0.0325245329207888 0.0273115627039723 0.0222571960309252 0.0173609241777604 0.0126221285742724 0.00804008281537994 0.0036139547554941]  distributed_mem=Block RAM  init_a=0  init_b=0  rst_a=off  rst_b=off  en_a=off  en_b=off  latency=2  write_mode_A=No Read On Write  write_mode_B=No Read On Write  dbl_ovrd=off  optimize=Area  block_type=dpram
  coeff_ram_4 : xbsIndex_r4/Dual Port RAM  depth=128  initVector=[-0.000657191316973142 -0.00477439242782273 -0.00873878459092812 -0.012551600410728 -0.0162141666163969 -0.019727901530796 -0.0230943124773524 -0.0263149931280691 -0.0293916207959064 -0.0323259536748176 -0.0351198280307586 -0.0377751553470205 -0.0402939194272665 -0.0426781734596743 -0.0449300370456098 -0.047051693196273 -0.0490453853007671 -0.0509134140690561 -0.0526581344532749 -0.0542819525508617 -0.0557873224929767 -0.0571767433216645 -0.0584527558592096 -0.0596179395731148 -0.0606749094401188 -0.0616263128126458 -0.0624748262910513 -0.063223152605004 -0.0638740175073049 -0.0644301666834137 -0.0648943626799071 -0.0652693818550541 -0.065558011354644 -0.0657630461161535 -0.0658872859042851 -0.0659335323808523 -0.0659045862119284 -0.0658032442151109 -0.0656322965496882 -0.0653945239524294 -0.0650926950216406 -0.0647295635520648 -0.0643078659231182 -0.0638303185428824 -0.0632996153501883 -0.0627184253770423 -0.0620893903735633 -0.0614151224975079 -0.0606982020703725 -0.0599411754019697 -0.0591465526852841 -0.0583168059633135 -0.0574543671695127 -0.0565616262433502 -0.0556409293223982 -0.054694577012269 -0.0537248227356155 -0.0527338711613074 -0.0517238767147951 -0.0506969421705693 -0.0496551173275191 -0.0486003977678913 -0.0475347237004437 -0.046459978888287 -0.045377989661801 -0.0442905240169085 -0.0431992907988871 -0.0421059389717933 -0.0410120569734727 -0.0399191721560267 -0.0388287503115035 -0.0377421952824818 -0.0366608486571164 -0.0355859895481129 -0.0345188344550056 -0.0334605372090128 -0.0324121889996507 -0.0313748184821953 -0.030349391964985 -0.0293368136754738 -0.0283379261038504 -0.0273535104229581 -0.0263842869831605 -0.0254309158807219 -0.0244939975981876 -0.023574073715173 -0.0226716276878965 -0.0217870856957163 -0.020920817552864 -0.0200731376834977 -0.0192443061581351 -0.0184345297894626 -0.0176439632854602 -0.0168727104577216 -0.0161208254828009 -0.0153883142143612 -0.0146751355438575 -0.0139812028074397 -0.0133063852367201 -0.0126505094510144 -0.0120133609886256 -0.0113946858747139 -0.0107941922232638 -0.0102115518706338 -0.0096464020381565 -0.00909834702123256 -0.00856695990235157 -0.00805178428545742 -0.00755233604906878 -0.00706810511555924 -0.00659855723399936 -0.00614313577396419 -0.00570126352771395 -0.00527234451816322 -0.00485576581006449 -0.00445089932184634 -0.00405710363556307 -0.00367372580243302 -0.00330010314146634 -0.00293556502870873 -0.00257943467465752 -0.00223103088743825 -0.00188966981936548 -0.00155466669454894 -0.00122533751524759 -0.000901000744716674 -0.000580978964339953 -0.000264600502887005]  distributed_mem=Block RAM  init_a=0  init_b=0  rst_a=off  rst_b=off  en_a=off  en_b=off  latency=2  write_mode_A=No Read On Write  write_mode_B=No Read On Write  dbl_ovrd=off  optimize=Area  block_type=dpram
  const_din : xbsIndex_r4/Constant  const=0  gui_display_data_type=Fixed-point  arith_type=Signed (2's comp)  n_bits=18  bin_pt=17  preci_type=Single  exp_width=8  frac_width=24  explicit_period=on  period=1  equ=P=C  opselect=C  inp2=PCIN>>17  opr=+  inp1=P  carry=CIN  dbl_ovrd=off  block_type=constant
  const_we : xbsIndex_r4/Constant  const=0  gui_display_data_type=Fixed-point  arith_type=Boolean  n_bits=16  bin_pt=14  preci_type=Single  exp_width=8  frac_width=24  explicit_period=on  period=1  equ=P=C  opselect=C  inp2=PCIN>>17  opr=+  inp1=P  carry=CIN  dbl_ovrd=off  block_type=constant
  cram_lower : Subsystem
  cram_upper : Subsystem
  sync_out : Outport
  coeff : Outport  Port=2
  coeff_rev : Outport  Port=3
  wires:
  sync/1 -> Counter1/1, Counter0/1, sync_out/1
  Counter0/1 -> coeff_ram_4/1, coeff_ram_3/1, Delay12/1, Delay11/1
  const_din/1 -> coeff_ram_4/5, coeff_ram_4/2, coeff_ram_3/5, coeff_ram_3/2, coeff_ram_2/5, coeff_ram_2/2, coeff_ram_1/5, coeff_ram_1/2
  const_we/1 -> coeff_ram_4/6, coeff_ram_4/3, coeff_ram_3/6, coeff_ram_3/3, coeff_ram_2/6, coeff_ram_2/3, coeff_ram_1/6, coeff_ram_1/3
  Counter1/1 -> Delay14/1, Delay13/1, coeff_ram_2/4, coeff_ram_1/4
  cram_lower/1(out) -> coeff_rev/1
  cram_upper/1(out) -> coeff/1
  coeff_ram_1/2 -> cram_lower/4(in3)
  coeff_ram_2/2 -> cram_lower/3(in2)
  coeff_ram_3/2 -> cram_lower/2(in1)
  coeff_ram_4/2 -> cram_lower/1(in0)
  coeff_ram_4/1 -> cram_upper/4(in3)
  coeff_ram_3/1 -> cram_upper/3(in2)
  coeff_ram_2/1 -> cram_upper/2(in1)
  coeff_ram_1/1 -> cram_upper/1(in0)
  Delay14/1 -> coeff_ram_4/4
  Delay13/1 -> coeff_ram_3/4
  Delay12/1 -> coeff_ram_2/1
  Delay11/1 -> coeff_ram_1/1
